simulink model "VirtualADAPT"  (Simulink 8.9)
settings: Solver=ode45  StartTime=0.0  StopTime=inf  RelTol=1e-3  EnableMultiTasking=on
PostLoadFcn:
  | FaultInjectionGUIHandle = FaultInjectionGUI;
InitFcn:
  | VirtualADAPTDataStructure
undefined symbols (not defined in the file or in its folder): ln
libraries: simulink x363
(part 21 of 26)

== system VirtualADAPT/VirtualADAPTv1/PowerStorage/Battery2Subsystem/RelayEY241/Sensor/R ==
  In : Inport
  Bond : Constant
  Computing R : Subsystem
  Fault Profile : Constant  Value=FP
  JuncDB : Constant  Value=2
  Junction Type : Constant  Value=0
  Ramp : simulink/Sources/Ramp  slope=FM  start=FT  X0=R
  Resistor Value : Constant  Value=R
  Step : Step  Time=FT  Before=R  After=(1 + FM)*R  SampleTime=0
  Out : Outport
  R : Outport  Port=2
  wires:
  Computing R/1(Out1) -> Out/1
  In/1 -> Computing R/1(In1)
  Resistor Value/1 -> Computing R/2(RNominal)
  Fault Profile/1 -> Computing R/3(Fault Profile)
  Step/1 -> Computing R/4(Step)
  Ramp/1 -> Computing R/5(Ramp)
  JuncDB/1 -> Computing R/6(JuncDB)
  Bond/1 -> Computing R/7(Bond)
  Junction Type/1 -> Computing R/8(Junction Type)
  Computing R/2(Out2) -> R/1

== system VirtualADAPT/VirtualADAPTv1/PowerStorage/Battery2Subsystem/RelayEY241/Sensor/TrueValueMod ==
  u(1) : Inport
  Function : Fcn  Expr=u(1)
  Mux : Mux  Inputs=1
  y(1) : Outport
  wires:
  u(1)/1 -> Mux/1
  Mux/1 -> Function/1
  Function/1 -> y(1)/1

== system VirtualADAPT/VirtualADAPTv1/PowerStorage/Battery2Subsystem/RelayEY241/Sensor/Value ==
  u(1) : Inport
  u(2) : Inport  Port=2
  Adder : Sum  Inputs=-  SaturateOnIntegerOverflow=on  InputSameDT=on  OutDataTypeStr=Inherit: Same as first input
  Gain : Gain  Gain=-1  SaturateOnIntegerOverflow=on  OutDataTypeStr=Inherit: Same as input  ParamDataTypeStr=Inherit: Same as input
  y(1) : Outport
  y(2) : Outport  Port=2
  O : Outport  Port=3
  wires:
  Adder/1 -> Gain/1
  u(2)/1 -> y(1)/1, O/1
  u(1)/1 -> Adder/1
  Gain/1 -> y(2)/1

== system VirtualADAPT/VirtualADAPTv1/PowerStorage/Battery2Subsystem/RelayEY244/ParallelConn/determineState ==
  In1 : Inport
  In2 : Inport  Port=2
  Fcn : Fcn  Expr=(!u(1)&&u(2)) || (u(1)&&(!u(3)))
  Memory : Memory
  Mux : Mux  Inputs=3
  Out1 : Outport
  wires:
  Memory/1 -> Mux/1
  In1/1 -> Mux/2
  In2/1 -> Mux/3
  Mux/1 -> Fcn/1
  Fcn/1 -> Memory/1, Out1/1

== system VirtualADAPT/VirtualADAPTv1/PowerStorage/Battery2Subsystem/RelayEY244/Relay/determineState ==
  In1 : Inport
  In2 : Inport  Port=2
  Fcn : Fcn  Expr=(!u(1)&&u(2)) || (u(1)&&(!u(3)))
  Memory : Memory
  Mux : Mux  Inputs=3
  Out1 : Outport
  wires:
  Memory/1 -> Mux/1
  In1/1 -> Mux/2
  In2/1 -> Mux/3
  Mux/1 -> Fcn/1
  Fcn/1 -> Memory/1, Out1/1

== system VirtualADAPT/VirtualADAPTv1/PowerStorage/Battery2Subsystem/RelayEY244/Sensor/MSe ==
  F : Inport
  Se : Inport  Port=2
  Bias : Step  Time=FT  After=FM  SampleTime=0
  Fault Inject : Subsystem
  Fault Profile : Constant  Value=FP
  Mult2 : Product  SaturateOnIntegerOverflow=on  RndMeth=Zero  InputSameDT=on  OutDataTypeStr=Inherit: Same as first input
  Multiply : Product  SaturateOnIntegerOverflow=on  RndMeth=Zero  InputSameDT=on  OutDataTypeStr=Inherit: Same as first input
  Ramp : simulink/Sources/Ramp  slope=FM  start=FT  X0=0
  Step : Step  Time=FT  Before=1  After=(1 + FM)  SampleTime=0
  StuckAt : Step  Time=FT  Before=1  After=0  SampleTime=0
  StuckAt2 : Step  Time=FT  After=FM  SampleTime=0
  Sum : Sum  SaturateOnIntegerOverflow=on  InputSameDT=on  OutDataTypeStr=Inherit: Same as first input
  Sum2 : Sum  SaturateOnIntegerOverflow=on  InputSameDT=on  OutDataTypeStr=Inherit: Same as first input
  Sum3 : Sum  SaturateOnIntegerOverflow=on  InputSameDT=on  OutDataTypeStr=Inherit: Same as first input
  E : Outport
  wires:
  Fault Inject/1(Out1) -> E/1
  Se/1 -> Fault Inject/1(Nominal), Multiply/1, Sum/1, Sum2/1, Mult2/1
  Fault Profile/1 -> Fault Inject/2(Fault Profile)
  Step/1 -> Multiply/2
  Ramp/1 -> Sum/2
  Multiply/1 -> Fault Inject/3(Step)
  Sum/1 -> Fault Inject/4(Ramp)
  Bias/1 -> Sum2/2
  Sum2/1 -> Fault Inject/5(Bias)
  StuckAt/1 -> Mult2/2
  Mult2/1 -> Sum3/1
  StuckAt2/1 -> Sum3/2
  Sum3/1 -> Fault Inject/6(stuckAt)

== system VirtualADAPT/VirtualADAPTv1/PowerStorage/Battery2Subsystem/RelayEY244/Sensor/R ==
  In : Inport
  Bond : Constant
  Computing R : Subsystem
  Fault Profile : Constant  Value=FP
  JuncDB : Constant  Value=2
  Junction Type : Constant  Value=0
  Ramp : simulink/Sources/Ramp  slope=FM  start=FT  X0=R
  Resistor Value : Constant  Value=R
  Step : Step  Time=FT  Before=R  After=(1 + FM)*R  SampleTime=0
  Out : Outport
  R : Outport  Port=2
  wires:
  Computing R/1(Out1) -> Out/1
  In/1 -> Computing R/1(In1)
  Resistor Value/1 -> Computing R/2(RNominal)
  Fault Profile/1 -> Computing R/3(Fault Profile)
  Step/1 -> Computing R/4(Step)
  Ramp/1 -> Computing R/5(Ramp)
  JuncDB/1 -> Computing R/6(JuncDB)
  Bond/1 -> Computing R/7(Bond)
  Junction Type/1 -> Computing R/8(Junction Type)
  Computing R/2(Out2) -> R/1

== system VirtualADAPT/VirtualADAPTv1/PowerStorage/Battery2Subsystem/RelayEY244/Sensor/TrueValueMod ==
  u(1) : Inport
  Function : Fcn  Expr=u(1)
  Mux : Mux  Inputs=1
  y(1) : Outport
  wires:
  u(1)/1 -> Mux/1
  Mux/1 -> Function/1
  Function/1 -> y(1)/1

== system VirtualADAPT/VirtualADAPTv1/PowerStorage/Battery2Subsystem/RelayEY244/Sensor/Value ==
  u(1) : Inport
  u(2) : Inport  Port=2
  Adder : Sum  Inputs=-  SaturateOnIntegerOverflow=on  InputSameDT=on  OutDataTypeStr=Inherit: Same as first input
  Gain : Gain  Gain=-1  SaturateOnIntegerOverflow=on  OutDataTypeStr=Inherit: Same as input  ParamDataTypeStr=Inherit: Same as input
  y(1) : Outport
  y(2) : Outport  Port=2
  O : Outport  Port=3
  wires:
  Adder/1 -> Gain/1
  u(2)/1 -> y(1)/1, O/1
  u(1)/1 -> Adder/1
  Gain/1 -> y(2)/1

== system VirtualADAPT/VirtualADAPTv1/PowerStorage/Battery2Subsystem/SensorE235/MSe/Fault Inject ==
  Nominal : Inport
  Fault Profile : Inport  Port=2
  Step : Inport  Port=3
  Ramp : Inport  Port=4
  Bias : Inport  Port=5
  stuckAt : Inport  Port=6
  Switch1 : MultiPortSwitch  Inputs=5  SaturateOnIntegerOverflow=on  InputSameDT=on
  Out1 : Outport
  wires:
  Nominal/1 -> Switch1/2
  Fault Profile/1 -> Switch1/1
  Step/1 -> Switch1/3
  Ramp/1 -> Switch1/4
  Bias/1 -> Switch1/5
  stuckAt/1 -> Switch1/6
  Switch1/1 -> Out1/1

== system VirtualADAPT/VirtualADAPTv1/PowerStorage/Battery2Subsystem/SensorE235/R/Computing R ==
  In1 : Inport
  RNominal : Inport  Port=2
  Fault Profile : Inport  Port=3
  Step : Inport  Port=4
  Ramp : Inport  Port=5
  JuncDB : Inport  Port=6
  Bond : Inport  Port=7
  Junction Type : Inport  Port=8
  CalcCausality : Fcn  Expr=(u(1) == u(2) && u(3) ==1) || (u(1) !=u(2) && u(3) == 0)
  CalcOutput : Fcn  Expr=(u(1)*u(2)^u(3))
  MinusOne : Constant  Value=-1
  Mux1 : Mux  Inputs=3
  Mux2 : Mux  Inputs=3
  One : Constant
  Switch1 : MultiPortSwitch  SaturateOnIntegerOverflow=on  InputSameDT=on
  Switch2 : MultiPortSwitch  DataPortOrder=Zero-based contiguous  Inputs=2  zeroidx=on  SaturateOnIntegerOverflow=on  InputSameDT=on
  Out1 : Outport
  Out2 : Outport  Port=2
  wires:
  In1/1 -> Mux2/1
  Fault Profile/1 -> Switch1/1
  RNominal/1 -> Switch1/2
  Step/1 -> Switch1/3
  Ramp/1 -> Switch1/4
  Switch1/1 -> Out2/1, Mux2/2
  JuncDB/1 -> Mux1/1
  Bond/1 -> Mux1/2
  Junction Type/1 -> Mux1/3
  Mux1/1 -> CalcCausality/1
  CalcCausality/1 -> Switch2/1
  One/1 -> Switch2/2
  MinusOne/1 -> Switch2/3
  Switch2/1 -> Mux2/3
  Mux2/1 -> CalcOutput/1
  CalcOutput/1 -> Out1/1

== system VirtualADAPT/VirtualADAPTv1/PowerStorage/Battery2Subsystem/SensorE240/MSe/Fault Inject ==
  Nominal : Inport
  Fault Profile : Inport  Port=2
  Step : Inport  Port=3
  Ramp : Inport  Port=4
  Bias : Inport  Port=5
  stuckAt : Inport  Port=6
  Switch1 : MultiPortSwitch  Inputs=5  SaturateOnIntegerOverflow=on  InputSameDT=on
  Out1 : Outport
  wires:
  Nominal/1 -> Switch1/2
  Fault Profile/1 -> Switch1/1
  Step/1 -> Switch1/3
  Ramp/1 -> Switch1/4
  Bias/1 -> Switch1/5
  stuckAt/1 -> Switch1/6
  Switch1/1 -> Out1/1

== system VirtualADAPT/VirtualADAPTv1/PowerStorage/Battery2Subsystem/SensorE240/R/Computing R ==
  In1 : Inport
  RNominal : Inport  Port=2
  Fault Profile : Inport  Port=3
  Step : Inport  Port=4
  Ramp : Inport  Port=5
  JuncDB : Inport  Port=6
  Bond : Inport  Port=7
  Junction Type : Inport  Port=8
  CalcCausality : Fcn  Expr=(u(1) == u(2) && u(3) ==1) || (u(1) !=u(2) && u(3) == 0)
  CalcOutput : Fcn  Expr=(u(1)*u(2)^u(3))
  MinusOne : Constant  Value=-1
  Mux1 : Mux  Inputs=3
  Mux2 : Mux  Inputs=3
  One : Constant
  Switch1 : MultiPortSwitch  SaturateOnIntegerOverflow=on  InputSameDT=on
  Switch2 : MultiPortSwitch  DataPortOrder=Zero-based contiguous  Inputs=2  zeroidx=on  SaturateOnIntegerOverflow=on  InputSameDT=on
  Out1 : Outport
  Out2 : Outport  Port=2
  wires:
  In1/1 -> Mux2/1
  Fault Profile/1 -> Switch1/1
  RNominal/1 -> Switch1/2
  Step/1 -> Switch1/3
  Ramp/1 -> Switch1/4
  Switch1/1 -> Out2/1, Mux2/2
  JuncDB/1 -> Mux1/1
  Bond/1 -> Mux1/2
  Junction Type/1 -> Mux1/3
  Mux1/1 -> CalcCausality/1
  CalcCausality/1 -> Switch2/1
  One/1 -> Switch2/2
  MinusOne/1 -> Switch2/3
  Switch2/1 -> Mux2/3
  Mux2/1 -> CalcOutput/1
  CalcOutput/1 -> Out1/1

== system VirtualADAPT/VirtualADAPTv1/PowerStorage/Battery2Subsystem/SensorE242/MSe/Fault Inject ==
  Nominal : Inport
  Fault Profile : Inport  Port=2
  Step : Inport  Port=3
  Ramp : Inport  Port=4
  Bias : Inport  Port=5
  stuckAt : Inport  Port=6
  Switch1 : MultiPortSwitch  Inputs=5  SaturateOnIntegerOverflow=on  InputSameDT=on
  Out1 : Outport
  wires:
  Nominal/1 -> Switch1/2
  Fault Profile/1 -> Switch1/1
  Step/1 -> Switch1/3
  Ramp/1 -> Switch1/4
  Bias/1 -> Switch1/5
  stuckAt/1 -> Switch1/6
  Switch1/1 -> Out1/1

== system VirtualADAPT/VirtualADAPTv1/PowerStorage/Battery2Subsystem/SensorE242/R/Computing R ==
  In1 : Inport
  RNominal : Inport  Port=2
  Fault Profile : Inport  Port=3
  Step : Inport  Port=4
  Ramp : Inport  Port=5
  JuncDB : Inport  Port=6
  Bond : Inport  Port=7
  Junction Type : Inport  Port=8
  CalcCausality : Fcn  Expr=(u(1) == u(2) && u(3) ==1) || (u(1) !=u(2) && u(3) == 0)
  CalcOutput : Fcn  Expr=(u(1)*u(2)^u(3))
  MinusOne : Constant  Value=-1
  Mux1 : Mux  Inputs=3
  Mux2 : Mux  Inputs=3
  One : Constant
  Switch1 : MultiPortSwitch  SaturateOnIntegerOverflow=on  InputSameDT=on
  Switch2 : MultiPortSwitch  DataPortOrder=Zero-based contiguous  Inputs=2  zeroidx=on  SaturateOnIntegerOverflow=on  InputSameDT=on
  Out1 : Outport
  Out2 : Outport  Port=2
  wires:
  In1/1 -> Mux2/1
  Fault Profile/1 -> Switch1/1
  RNominal/1 -> Switch1/2
  Step/1 -> Switch1/3
  Ramp/1 -> Switch1/4
  Switch1/1 -> Out2/1, Mux2/2
  JuncDB/1 -> Mux1/1
  Bond/1 -> Mux1/2
  Junction Type/1 -> Mux1/3
  Mux1/1 -> CalcCausality/1
  CalcCausality/1 -> Switch2/1
  One/1 -> Switch2/2
  MinusOne/1 -> Switch2/3
  Switch2/1 -> Mux2/3
  Mux2/1 -> CalcOutput/1
  CalcOutput/1 -> Out1/1

== system VirtualADAPT/VirtualADAPTv1/PowerStorage/Battery2Subsystem/SensorISH236/MSe/Fault Inject ==
  Nominal : Inport
  Fault Profile : Inport  Port=2
  Step : Inport  Port=3
  Ramp : Inport  Port=4
  Bias : Inport  Port=5
  stuckAt : Inport  Port=6
  Switch1 : MultiPortSwitch  Inputs=5  SaturateOnIntegerOverflow=on  InputSameDT=on
  Out1 : Outport
  wires:
  Nominal/1 -> Switch1/2
  Fault Profile/1 -> Switch1/1
  Step/1 -> Switch1/3
  Ramp/1 -> Switch1/4
  Bias/1 -> Switch1/5
  stuckAt/1 -> Switch1/6
  Switch1/1 -> Out1/1

== system VirtualADAPT/VirtualADAPTv1/PowerStorage/Battery2Subsystem/SensorISH236/R/Computing R ==
  In1 : Inport
  RNominal : Inport  Port=2
  Fault Profile : Inport  Port=3
  Step : Inport  Port=4
  Ramp : Inport  Port=5
  JuncDB : Inport  Port=6
  Bond : Inport  Port=7
  Junction Type : Inport  Port=8
  CalcCausality : Fcn  Expr=(u(1) == u(2) && u(3) ==1) || (u(1) !=u(2) && u(3) == 0)
  CalcOutput : Fcn  Expr=(u(1)*u(2)^u(3))
  MinusOne : Constant  Value=-1
  Mux1 : Mux  Inputs=3
  Mux2 : Mux  Inputs=3
  One : Constant
  Switch1 : MultiPortSwitch  SaturateOnIntegerOverflow=on  InputSameDT=on
  Switch2 : MultiPortSwitch  DataPortOrder=Zero-based contiguous  Inputs=2  zeroidx=on  SaturateOnIntegerOverflow=on  InputSameDT=on
  Out1 : Outport
  Out2 : Outport  Port=2
  wires:
  In1/1 -> Mux2/1
  Fault Profile/1 -> Switch1/1
  RNominal/1 -> Switch1/2
  Step/1 -> Switch1/3
  Ramp/1 -> Switch1/4
  Switch1/1 -> Out2/1, Mux2/2
  JuncDB/1 -> Mux1/1
  Bond/1 -> Mux1/2
  Junction Type/1 -> Mux1/3
  Mux1/1 -> CalcCausality/1
  CalcCausality/1 -> Switch2/1
  One/1 -> Switch2/2
  MinusOne/1 -> Switch2/3
  Switch2/1 -> Mux2/3
  Mux2/1 -> CalcOutput/1
  CalcOutput/1 -> Out1/1

== system VirtualADAPT/VirtualADAPTv1/PowerStorage/Battery2Subsystem/SensorIT240/MSe/Fault Inject ==
  Nominal : Inport
  Fault Profile : Inport  Port=2
  Step : Inport  Port=3
  Ramp : Inport  Port=4
  Bias : Inport  Port=5
  stuckAt : Inport  Port=6
  Switch1 : MultiPortSwitch  Inputs=5  SaturateOnIntegerOverflow=on  InputSameDT=on
  Out1 : Outport
  wires:
  Nominal/1 -> Switch1/2
  Fault Profile/1 -> Switch1/1
  Step/1 -> Switch1/3
  Ramp/1 -> Switch1/4
  Bias/1 -> Switch1/5
  stuckAt/1 -> Switch1/6
  Switch1/1 -> Out1/1

== system VirtualADAPT/VirtualADAPTv1/PowerStorage/Battery2Subsystem/SensorIT240/R/Computing R ==
  In1 : Inport
  RNominal : Inport  Port=2
  Fault Profile : Inport  Port=3
  Step : Inport  Port=4
  Ramp : Inport  Port=5
  JuncDB : Inport  Port=6
  Bond : Inport  Port=7
  Junction Type : Inport  Port=8
  CalcCausality : Fcn  Expr=(u(1) == u(2) && u(3) ==1) || (u(1) !=u(2) && u(3) == 0)
  CalcOutput : Fcn  Expr=(u(1)*u(2)^u(3))
  MinusOne : Constant  Value=-1
  Mux1 : Mux  Inputs=3
  Mux2 : Mux  Inputs=3
  One : Constant
  Switch1 : MultiPortSwitch  SaturateOnIntegerOverflow=on  InputSameDT=on
  Switch2 : MultiPortSwitch  DataPortOrder=Zero-based contiguous  Inputs=2  zeroidx=on  SaturateOnIntegerOverflow=on  InputSameDT=on
  Out1 : Outport
  Out2 : Outport  Port=2
  wires:
  In1/1 -> Mux2/1
  Fault Profile/1 -> Switch1/1
  RNominal/1 -> Switch1/2
  Step/1 -> Switch1/3
  Ramp/1 -> Switch1/4
  Switch1/1 -> Out2/1, Mux2/2
  JuncDB/1 -> Mux1/1
  Bond/1 -> Mux1/2
  Junction Type/1 -> Mux1/3
  Mux1/1 -> CalcCausality/1
  CalcCausality/1 -> Switch2/1
  One/1 -> Switch2/2
  MinusOne/1 -> Switch2/3
  Switch2/1 -> Mux2/3
  Mux2/1 -> CalcOutput/1
  CalcOutput/1 -> Out1/1

== system VirtualADAPT/VirtualADAPTv1/PowerStorage/Battery2Subsystem/SensorTE228/MSe/Fault Inject ==
  Nominal : Inport
  Fault Profile : Inport  Port=2
  Step : Inport  Port=3
  Ramp : Inport  Port=4
  Bias : Inport  Port=5
  stuckAt : Inport  Port=6
  Switch1 : MultiPortSwitch  Inputs=5  SaturateOnIntegerOverflow=on  InputSameDT=on
  Out1 : Outport
  wires:
  Nominal/1 -> Switch1/2
  Fault Profile/1 -> Switch1/1
  Step/1 -> Switch1/3
  Ramp/1 -> Switch1/4
  Bias/1 -> Switch1/5
  stuckAt/1 -> Switch1/6
  Switch1/1 -> Out1/1

== system VirtualADAPT/VirtualADAPTv1/PowerStorage/Battery2Subsystem/SensorTE228/R/Computing R ==
  In1 : Inport
  RNominal : Inport  Port=2
  Fault Profile : Inport  Port=3
  Step : Inport  Port=4
  Ramp : Inport  Port=5
  JuncDB : Inport  Port=6
  Bond : Inport  Port=7
  Junction Type : Inport  Port=8
  CalcCausality : Fcn  Expr=(u(1) == u(2) && u(3) ==1) || (u(1) !=u(2) && u(3) == 0)
  CalcOutput : Fcn  Expr=(u(1)*u(2)^u(3))
  MinusOne : Constant  Value=-1
  Mux1 : Mux  Inputs=3
  Mux2 : Mux  Inputs=3
  One : Constant
  Switch1 : MultiPortSwitch  SaturateOnIntegerOverflow=on  InputSameDT=on
  Switch2 : MultiPortSwitch  DataPortOrder=Zero-based contiguous  Inputs=2  zeroidx=on  SaturateOnIntegerOverflow=on  InputSameDT=on
  Out1 : Outport
  Out2 : Outport  Port=2
  wires:
  In1/1 -> Mux2/1
  Fault Profile/1 -> Switch1/1
  RNominal/1 -> Switch1/2
  Step/1 -> Switch1/3
  Ramp/1 -> Switch1/4
  Switch1/1 -> Out2/1, Mux2/2
  JuncDB/1 -> Mux1/1
  Bond/1 -> Mux1/2
  Junction Type/1 -> Mux1/3
  Mux1/1 -> CalcCausality/1
  CalcCausality/1 -> Switch2/1
  One/1 -> Switch2/2
  MinusOne/1 -> Switch2/3
  Switch2/1 -> Mux2/3
  Mux2/1 -> CalcOutput/1
  CalcOutput/1 -> Out1/1

== system VirtualADAPT/VirtualADAPTv1/PowerStorage/Battery2Subsystem/SensorTE228/TempBias/Fault Inject ==
  Nominal : Inport
  Fault Profile : Inport  Port=2
  Step : Inport  Port=3
  Ramp : Inport  Port=4
  Bias : Inport  Port=5
  stuckAt : Inport  Port=6
  Switch1 : MultiPortSwitch  Inputs=5  SaturateOnIntegerOverflow=on  InputSameDT=on
  Out1 : Outport
  wires:
  Nominal/1 -> Switch1/2
  Fault Profile/1 -> Switch1/1
  Step/1 -> Switch1/3
  Ramp/1 -> Switch1/4
  Bias/1 -> Switch1/5
  stuckAt/1 -> Switch1/6
  Switch1/1 -> Out1/1

== system VirtualADAPT/VirtualADAPTv1/PowerStorage/Battery2Subsystem/SensorTE229/MSe/Fault Inject ==
  Nominal : Inport
  Fault Profile : Inport  Port=2
  Step : Inport  Port=3
  Ramp : Inport  Port=4
  Bias : Inport  Port=5
  stuckAt : Inport  Port=6
  Switch1 : MultiPortSwitch  Inputs=5  SaturateOnIntegerOverflow=on  InputSameDT=on
  Out1 : Outport
  wires:
  Nominal/1 -> Switch1/2
  Fault Profile/1 -> Switch1/1
  Step/1 -> Switch1/3
  Ramp/1 -> Switch1/4
  Bias/1 -> Switch1/5
  stuckAt/1 -> Switch1/6
  Switch1/1 -> Out1/1

== system VirtualADAPT/VirtualADAPTv1/PowerStorage/Battery2Subsystem/SensorTE229/R/Computing R ==
  In1 : Inport
  RNominal : Inport  Port=2
  Fault Profile : Inport  Port=3
  Step : Inport  Port=4
  Ramp : Inport  Port=5
  JuncDB : Inport  Port=6
  Bond : Inport  Port=7
  Junction Type : Inport  Port=8
  CalcCausality : Fcn  Expr=(u(1) == u(2) && u(3) ==1) || (u(1) !=u(2) && u(3) == 0)
  CalcOutput : Fcn  Expr=(u(1)*u(2)^u(3))
  MinusOne : Constant  Value=-1
  Mux1 : Mux  Inputs=3
  Mux2 : Mux  Inputs=3
  One : Constant
  Switch1 : MultiPortSwitch  SaturateOnIntegerOverflow=on  InputSameDT=on
  Switch2 : MultiPortSwitch  DataPortOrder=Zero-based contiguous  Inputs=2  zeroidx=on  SaturateOnIntegerOverflow=on  InputSameDT=on
  Out1 : Outport
  Out2 : Outport  Port=2
  wires:
  In1/1 -> Mux2/1
  Fault Profile/1 -> Switch1/1
  RNominal/1 -> Switch1/2
  Step/1 -> Switch1/3
  Ramp/1 -> Switch1/4
  Switch1/1 -> Out2/1, Mux2/2
  JuncDB/1 -> Mux1/1
  Bond/1 -> Mux1/2
  Junction Type/1 -> Mux1/3
  Mux1/1 -> CalcCausality/1
  CalcCausality/1 -> Switch2/1
  One/1 -> Switch2/2
  MinusOne/1 -> Switch2/3
  Switch2/1 -> Mux2/3
  Mux2/1 -> CalcOutput/1
  CalcOutput/1 -> Out1/1

== system VirtualADAPT/VirtualADAPTv1/PowerStorage/Battery2Subsystem/SensorTE229/TempBias/Fault Inject ==
  Nominal : Inport
  Fault Profile : Inport  Port=2
  Step : Inport  Port=3
  Ramp : Inport  Port=4
  Bias : Inport  Port=5
  stuckAt : Inport  Port=6
  Switch1 : MultiPortSwitch  Inputs=5  SaturateOnIntegerOverflow=on  InputSameDT=on
  Out1 : Outport
  wires:
  Nominal/1 -> Switch1/2
  Fault Profile/1 -> Switch1/1
  Step/1 -> Switch1/3
  Ramp/1 -> Switch1/4
  Bias/1 -> Switch1/5
  stuckAt/1 -> Switch1/6
  Switch1/1 -> Out1/1

== system VirtualADAPT/VirtualADAPTv1/PowerStorage/RelayEY160/Sensor/MSe/Fault Inject ==
  Nominal : Inport
  Fault Profile : Inport  Port=2
  Step : Inport  Port=3
  Ramp : Inport  Port=4
  Bias : Inport  Port=5
  stuckAt : Inport  Port=6
  Switch1 : MultiPortSwitch  Inputs=5  SaturateOnIntegerOverflow=on  InputSameDT=on
  Out1 : Outport
  wires:
  Nominal/1 -> Switch1/2
  Fault Profile/1 -> Switch1/1
  Step/1 -> Switch1/3
  Ramp/1 -> Switch1/4
  Bias/1 -> Switch1/5
  stuckAt/1 -> Switch1/6
  Switch1/1 -> Out1/1

== system VirtualADAPT/VirtualADAPTv1/PowerStorage/RelayEY160/Sensor/R/Computing R ==
  In1 : Inport
  RNominal : Inport  Port=2
  Fault Profile : Inport  Port=3
  Step : Inport  Port=4
  Ramp : Inport  Port=5
  JuncDB : Inport  Port=6
  Bond : Inport  Port=7
  Junction Type : Inport  Port=8
  CalcCausality : Fcn  Expr=(u(1) == u(2) && u(3) ==1) || (u(1) !=u(2) && u(3) == 0)
  CalcOutput : Fcn  Expr=(u(1)*u(2)^u(3))
  MinusOne : Constant  Value=-1
  Mux1 : Mux  Inputs=3
  Mux2 : Mux  Inputs=3
  One : Constant
  Switch1 : MultiPortSwitch  SaturateOnIntegerOverflow=on  InputSameDT=on
  Switch2 : MultiPortSwitch  DataPortOrder=Zero-based contiguous  Inputs=2  zeroidx=on  SaturateOnIntegerOverflow=on  InputSameDT=on
  Out1 : Outport
  Out2 : Outport  Port=2
  wires:
  In1/1 -> Mux2/1
  Fault Profile/1 -> Switch1/1
  RNominal/1 -> Switch1/2
  Step/1 -> Switch1/3
  Ramp/1 -> Switch1/4
  Switch1/1 -> Out2/1, Mux2/2
  JuncDB/1 -> Mux1/1
  Bond/1 -> Mux1/2
  Junction Type/1 -> Mux1/3
  Mux1/1 -> CalcCausality/1
  CalcCausality/1 -> Switch2/1
  One/1 -> Switch2/2
  MinusOne/1 -> Switch2/3
  Switch2/1 -> Mux2/3
  Mux2/1 -> CalcOutput/1
  CalcOutput/1 -> Out1/1

== system VirtualADAPT/VirtualADAPTv1/PowerStorage/RelayEY260/Sensor/MSe/Fault Inject ==
  Nominal : Inport
  Fault Profile : Inport  Port=2
  Step : Inport  Port=3
  Ramp : Inport  Port=4
  Bias : Inport  Port=5
  stuckAt : Inport  Port=6
  Switch1 : MultiPortSwitch  Inputs=5  SaturateOnIntegerOverflow=on  InputSameDT=on
  Out1 : Outport
  wires:
  Nominal/1 -> Switch1/2
  Fault Profile/1 -> Switch1/1
  Step/1 -> Switch1/3
  Ramp/1 -> Switch1/4
  Bias/1 -> Switch1/5
  stuckAt/1 -> Switch1/6
  Switch1/1 -> Out1/1

== system VirtualADAPT/VirtualADAPTv1/PowerStorage/RelayEY260/Sensor/R/Computing R ==
  In1 : Inport
  RNominal : Inport  Port=2
  Fault Profile : Inport  Port=3
  Step : Inport  Port=4
  Ramp : Inport  Port=5
  JuncDB : Inport  Port=6
  Bond : Inport  Port=7
  Junction Type : Inport  Port=8
  CalcCausality : Fcn  Expr=(u(1) == u(2) && u(3) ==1) || (u(1) !=u(2) && u(3) == 0)
  CalcOutput : Fcn  Expr=(u(1)*u(2)^u(3))
  MinusOne : Constant  Value=-1
  Mux1 : Mux  Inputs=3
  Mux2 : Mux  Inputs=3
  One : Constant
  Switch1 : MultiPortSwitch  SaturateOnIntegerOverflow=on  InputSameDT=on
  Switch2 : MultiPortSwitch  DataPortOrder=Zero-based contiguous  Inputs=2  zeroidx=on  SaturateOnIntegerOverflow=on  InputSameDT=on
  Out1 : Outport
  Out2 : Outport  Port=2
  wires:
  In1/1 -> Mux2/1
  Fault Profile/1 -> Switch1/1
  RNominal/1 -> Switch1/2
  Step/1 -> Switch1/3
  Ramp/1 -> Switch1/4
  Switch1/1 -> Out2/1, Mux2/2
  JuncDB/1 -> Mux1/1
  Bond/1 -> Mux1/2
  Junction Type/1 -> Mux1/3
  Mux1/1 -> CalcCausality/1
  CalcCausality/1 -> Switch2/1
  One/1 -> Switch2/2
  MinusOne/1 -> Switch2/3
  Switch2/1 -> Mux2/3
  Mux2/1 -> CalcOutput/1
  CalcOutput/1 -> Out1/1

== system VirtualADAPT/VirtualADAPTv1/PowerDistribution/ACLoadBank1/L1A/LGT6/LightBulb1/ComputePower ==
  u(1) : Inport
  u(2) : Inport  Port=2
  Function : Fcn  Expr=abs(u(1)*u(2))
  Mux : Mux  Inputs=2
  y(1) : Outport
  wires:
  u(1)/1 -> Mux/1
  u(2)/1 -> Mux/2
  Mux/1 -> Function/1
  Function/1 -> y(1)/1

== system VirtualADAPT/VirtualADAPTv1/PowerDistribution/ACLoadBank1/L1A/LGT6/LightBulb1/I ==
  u(1) : Inport
  u(2) : Inport  Port=2
  Adder : Sum  Inputs=+  SaturateOnIntegerOverflow=on  InputSameDT=on  OutDataTypeStr=Inherit: Same as first input
  Gain : Gain  SaturateOnIntegerOverflow=on  OutDataTypeStr=Inherit: Same as input  ParamDataTypeStr=Inherit: Same as input
  y(1) : Outport
  y(2) : Outport  Port=2
  O : Outport  Port=3
  wires:
  Adder/1 -> Gain/1
  Gain/1 -> y(1)/1
  u(1)/1 -> y(2)/1, O/1
  u(2)/1 -> Adder/1

== system VirtualADAPT/VirtualADAPTv1/PowerDistribution/ACLoadBank1/L1A/LGT6/LightBulb1/R ==
  In : Inport
  Bond : Constant  Value=2
  Computing R : Subsystem
  Fault Profile : Constant  Value=FP
  JuncDB : Constant
  Junction Type : Constant  Value=0
  Ramp : simulink/Sources/Ramp  slope=FM  start=FT  X0=R
  Resistor Value : Constant  Value=R
  Step : Step  Time=FT  Before=R  After=120.5/(FM+120.5/R)  SampleTime=0
  Out : Outport
  R : Outport  Port=2
  wires:
  Computing R/1(Out1) -> Out/1
  In/1 -> Computing R/1(In1)
  Resistor Value/1 -> Computing R/2(RNominal)
  Fault Profile/1 -> Computing R/3(Fault Profile)
  Step/1 -> Computing R/4(Step)
  Ramp/1 -> Computing R/5(Ramp)
  JuncDB/1 -> Computing R/6(JuncDB)
  Bond/1 -> Computing R/7(Bond)
  Junction Type/1 -> Computing R/8(Junction Type)
  Computing R/2(Out2) -> R/1

== system VirtualADAPT/VirtualADAPTv1/PowerDistribution/ACLoadBank1/L1A/LGT6/LightBulb1/TemperatureModel ==
  AmbientTemp : Inport
  Power : Inport  Port=2
  AmbTemp : Subsystem  mask{FP=Nominal, FT=0, FM=0}
      mask prompts: FP "Fault Profile:"; FT "Fault Injection Time:"; FM "Fault Magnitude/Slope/Bias/StuckAtValue"
      mask TypeOptions FP: Nominal | Abrupt | Incipient | Bias | StuckAt
      mask Display: image(imread('MSe.png','BackgroundColor',[1 1 1]))
  Ctheta : Subsystem  mask{C=51.711, IC=22, FP=Nominal, FT=0, FM=0}
      mask prompts: C "Value of C:"; IC "Initial Condition:"; FP "Fault Profile:"; FT "Fault Injection Time:"; FM "Fault Magnitude/Slope"
      mask TypeOptions FP: Nominal | Abrupt | Incipient
      mask Display: image(imread('C.png','BackgroundColor',[1 1 1]))
  ModAmbTemp : Subsystem
      mask Display: image(imread('fx.png','BackgroundColor',[1 1 1]))
  ModPs : Subsystem
      mask Display: image(imread('fx.png','BackgroundColor',[1 1 1]))
  OJ : Subsystem
      mask Display: image(imread('1.png','BackgroundColor',[1 1 1]))
  Rtheta : Subsystem  mask{R=2.3686, FP=Nominal, FT=0, FM=0}
      mask prompts: R "Value of R:"; FP "Fault Profile:"; FT "Fault Injection Time:"; FM "Fault Magnitude/Slope"
      mask TypeOptions FP: Nominal | Abrupt | Incipient
      mask Display: image(imread('R.png','BackgroundColor',[1 1 1]))
  SfPs : Subsystem  mask{FP=Nominal, FT=0, FM=0}
      mask prompts: FP "Fault Profile:"; FT "Fault Injection Time:"; FM "Fault Magnitude/Slope/Bias/StuckAtValue"
      mask TypeOptions FP: Nominal | Abrupt | Incipient | Bias | StuckAt
      mask Display: image(imread('MSf.png','BackgroundColor',[1 1 1]))
  theta : Subsystem
      mask Display: image(imread('0.png','BackgroundColor',[1 1 1]))
  Temp : Outport
  wires:
  theta/1(y(1)) -> Ctheta/1(F)
  Ctheta/1(E) -> theta/1(u(1))
  OJ/1(y(1)) -> Rtheta/1(In)
  Rtheta/1(Out) -> OJ/1(u(1))
  theta/2(y(2)) -> OJ/2(u(2))
  OJ/2(y(2)) -> theta/2(u(2))
  SfPs/1(F) -> theta/3(u(3))
  theta/3(y(3)) -> SfPs/1(E)
  AmbTemp/1(E) -> OJ/3(u(3))
  OJ/3(y(3)) -> AmbTemp/1(F)
  ModAmbTemp/1(y(1)) -> AmbTemp/2(Se)
  ModPs/1(y(1)) -> SfPs/2(Sf)
  Power/1 -> ModPs/1(u(1))
  AmbientTemp/1 -> ModAmbTemp/1(u(1))
  theta/4(O) -> Temp/1

== system VirtualADAPT/VirtualADAPTv1/PowerDistribution/ACLoadBank1/L1A/LGT6/LightBulb1/V ==
  u(1) : Inport
  u(2) : Inport  Port=2
  Adder : Sum  Inputs=-  SaturateOnIntegerOverflow=on  InputSameDT=on  OutDataTypeStr=Inherit: Same as first input
  Gain : Gain  Gain=-1  SaturateOnIntegerOverflow=on  OutDataTypeStr=Inherit: Same as input  ParamDataTypeStr=Inherit: Same as input
  y(1) : Outport
  y(2) : Outport  Port=2
  O : Outport  Port=3
  wires:
  Adder/1 -> Gain/1
  Gain/1 -> y(1)/1
  u(1)/1 -> y(2)/1, O/1
  u(2)/1 -> Adder/1

== system VirtualADAPT/VirtualADAPTv1/PowerDistribution/ACLoadBank1/L1A/LGT6/LightBulb2/ComputePower ==
  u(1) : Inport
  u(2) : Inport  Port=2
  Function : Fcn  Expr=abs(u(2)*u(1))
  Mux : Mux  Inputs=2
  y(1) : Outport
  wires:
  u(1)/1 -> Mux/1
  u(2)/1 -> Mux/2
  Mux/1 -> Function/1
  Function/1 -> y(1)/1

== system VirtualADAPT/VirtualADAPTv1/PowerDistribution/ACLoadBank1/L1A/LGT6/LightBulb2/I ==
  u(1) : Inport
  u(2) : Inport  Port=2
  Adder : Sum  Inputs=+  SaturateOnIntegerOverflow=on  InputSameDT=on  OutDataTypeStr=Inherit: Same as first input
  Gain : Gain  SaturateOnIntegerOverflow=on  OutDataTypeStr=Inherit: Same as input  ParamDataTypeStr=Inherit: Same as input
  y(1) : Outport
  y(2) : Outport  Port=2
  O : Outport  Port=3
  wires:
  Adder/1 -> Gain/1
  Gain/1 -> y(1)/1
  u(1)/1 -> y(2)/1, O/1
  u(2)/1 -> Adder/1

== system VirtualADAPT/VirtualADAPTv1/PowerDistribution/ACLoadBank1/L1A/LGT6/LightBulb2/R ==
  In : Inport
  Bond : Constant  Value=2
  Computing R : Subsystem
  Fault Profile : Constant  Value=FP
  JuncDB : Constant
  Junction Type : Constant  Value=0
  Ramp : simulink/Sources/Ramp  slope=FM  start=FT  X0=R
  Resistor Value : Constant  Value=R
  Step : Step  Time=FT  Before=R  After=120.5/(FM+120.5/R)  SampleTime=0
  Out : Outport
  R : Outport  Port=2
  wires:
  Computing R/1(Out1) -> Out/1
  In/1 -> Computing R/1(In1)
  Resistor Value/1 -> Computing R/2(RNominal)
  Fault Profile/1 -> Computing R/3(Fault Profile)
  Step/1 -> Computing R/4(Step)
  Ramp/1 -> Computing R/5(Ramp)
  JuncDB/1 -> Computing R/6(JuncDB)
  Bond/1 -> Computing R/7(Bond)
  Junction Type/1 -> Computing R/8(Junction Type)
  Computing R/2(Out2) -> R/1

== system VirtualADAPT/VirtualADAPTv1/PowerDistribution/ACLoadBank1/L1A/LGT6/LightBulb2/TemperatureModel ==
  AmbientTemp : Inport
  Power : Inport  Port=2
  AmbTemp : Subsystem  mask{FP=Nominal, FT=0, FM=0}
      mask prompts: FP "Fault Profile:"; FT "Fault Injection Time:"; FM "Fault Magnitude/Slope/Bias/StuckAtValue"
      mask TypeOptions FP: Nominal | Abrupt | Incipient | Bias | StuckAt
      mask Display: image(imread('MSe.png','BackgroundColor',[1 1 1]))
  Ctheta : Subsystem  mask{C=96.176, IC=22, FP=Nominal, FT=0, FM=0}
      mask prompts: C "Value of C:"; IC "Initial Condition:"; FP "Fault Profile:"; FT "Fault Injection Time:"; FM "Fault Magnitude/Slope"
      mask TypeOptions FP: Nominal | Abrupt | Incipient
      mask Display: image(imread('C.png','BackgroundColor',[1 1 1]))
  ModAmbTemp : Subsystem
      mask Display: image(imread('fx.png','BackgroundColor',[1 1 1]))
  ModPs : Subsystem
      mask Display: image(imread('fx.png','BackgroundColor',[1 1 1]))
  OJ : Subsystem
      mask Display: image(imread('1.png','BackgroundColor',[1 1 1]))
  Rtheta : Subsystem  mask{R=1.5203, FP=Nominal, FT=0, FM=0}
      mask prompts: R "Value of R:"; FP "Fault Profile:"; FT "Fault Injection Time:"; FM "Fault Magnitude/Slope"
      mask TypeOptions FP: Nominal | Abrupt | Incipient
      mask Display: image(imread('R.png','BackgroundColor',[1 1 1]))
  SfPs : Subsystem  mask{FP=Nominal, FT=0, FM=0}
      mask prompts: FP "Fault Profile:"; FT "Fault Injection Time:"; FM "Fault Magnitude/Slope/Bias/StuckAtValue"
      mask TypeOptions FP: Nominal | Abrupt | Incipient | Bias | StuckAt
      mask Display: image(imread('MSf.png','BackgroundColor',[1 1 1]))
  theta : Subsystem
      mask Display: image(imread('0.png','BackgroundColor',[1 1 1]))
  Temp : Outport
  wires:
  SfPs/1(F) -> theta/1(u(1))
  theta/1(y(1)) -> SfPs/1(E)
  theta/2(y(2)) -> OJ/1(u(1))
  OJ/1(y(1)) -> theta/2(u(2))
  theta/3(y(3)) -> Ctheta/1(F)
  Ctheta/1(E) -> theta/3(u(3))
  OJ/2(y(2)) -> Rtheta/1(In)
  Rtheta/1(Out) -> OJ/2(u(2))
  AmbTemp/1(E) -> OJ/3(u(3))
  OJ/3(y(3)) -> AmbTemp/1(F)
  ModAmbTemp/1(y(1)) -> AmbTemp/2(Se)
  ModPs/1(y(1)) -> SfPs/2(Sf)
  Power/1 -> ModPs/1(u(1))
  AmbientTemp/1 -> ModAmbTemp/1(u(1))
  theta/4(O) -> Temp/1

== system VirtualADAPT/VirtualADAPTv1/PowerDistribution/ACLoadBank1/L1A/LGT6/LightBulb2/V ==
  u(1) : Inport
  u(2) : Inport  Port=2
  Adder : Sum  Inputs=-  SaturateOnIntegerOverflow=on  InputSameDT=on  OutDataTypeStr=Inherit: Same as first input
  Gain : Gain  Gain=-1  SaturateOnIntegerOverflow=on  OutDataTypeStr=Inherit: Same as input  ParamDataTypeStr=Inherit: Same as input
  y(1) : Outport
  y(2) : Outport  Port=2
  O : Outport  Port=3
  wires:
  Adder/1 -> Gain/1
  Gain/1 -> y(1)/1
  u(1)/1 -> y(2)/1, O/1
  u(2)/1 -> Adder/1

== system VirtualADAPT/VirtualADAPTv1/PowerDistribution/ACLoadBank1/L1A/LGT6/LightBulb3/ComputePower ==
  u(1) : Inport
  u(2) : Inport  Port=2
  Function : Fcn  Expr=abs(u(1)*u(2))
  Mux : Mux  Inputs=2
  y(1) : Outport
  wires:
  u(1)/1 -> Mux/1
  u(2)/1 -> Mux/2
  Mux/1 -> Function/1
  Function/1 -> y(1)/1

== system VirtualADAPT/VirtualADAPTv1/PowerDistribution/ACLoadBank1/L1A/LGT6/LightBulb3/I ==
  u(1) : Inport
  u(2) : Inport  Port=2
  Adder : Sum  Inputs=+  SaturateOnIntegerOverflow=on  InputSameDT=on  OutDataTypeStr=Inherit: Same as first input
  Gain : Gain  SaturateOnIntegerOverflow=on  OutDataTypeStr=Inherit: Same as input  ParamDataTypeStr=Inherit: Same as input
  y(1) : Outport
  y(2) : Outport  Port=2
  O : Outport  Port=3
  wires:
  Adder/1 -> Gain/1
  Gain/1 -> y(1)/1
  u(1)/1 -> y(2)/1, O/1
  u(2)/1 -> Adder/1

== system VirtualADAPT/VirtualADAPTv1/PowerDistribution/ACLoadBank1/L1A/LGT6/LightBulb3/R ==
  In : Inport
  Bond : Constant
  Computing R : Subsystem
  Fault Profile : Constant  Value=FP
  JuncDB : Constant  Value=2
  Junction Type : Constant  Value=0
  Ramp : simulink/Sources/Ramp  slope=FM  start=FT  X0=R
  Resistor Value : Constant  Value=R
  Step : Step  Time=FT  Before=R  After=120.5/(FM+120.5/R)  SampleTime=0
  Out : Outport
  R : Outport  Port=2
  wires:
  Computing R/1(Out1) -> Out/1
  In/1 -> Computing R/1(In1)
  Resistor Value/1 -> Computing R/2(RNominal)
  Fault Profile/1 -> Computing R/3(Fault Profile)
  Step/1 -> Computing R/4(Step)
  Ramp/1 -> Computing R/5(Ramp)
  JuncDB/1 -> Computing R/6(JuncDB)
  Bond/1 -> Computing R/7(Bond)
  Junction Type/1 -> Computing R/8(Junction Type)
  Computing R/2(Out2) -> R/1

== system VirtualADAPT/VirtualADAPTv1/PowerDistribution/ACLoadBank1/L1A/LGT6/LightBulb3/TemperatureModel ==
  AmbientTemp : Inport
  Power : Inport  Port=2
  AmbTemp : Subsystem  mask{FP=Nominal, FT=0, FM=0}
      mask prompts: FP "Fault Profile:"; FT "Fault Injection Time:"; FM "Fault Magnitude/Slope/Bias/StuckAtValue"
      mask TypeOptions FP: Nominal | Abrupt | Incipient | Bias | StuckAt
      mask Display: image(imread('MSe.png','BackgroundColor',[1 1 1]))
  Ctheta : Subsystem  mask{C=104.07, IC=22, FP=Nominal, FT=0, FM=0}
      mask prompts: C "Value of C:"; IC "Initial Condition:"; FP "Fault Profile:"; FT "Fault Injection Time:"; FM "Fault Magnitude/Slope"
      mask TypeOptions FP: Nominal | Abrupt | Incipient
      mask Display: image(imread('C.png','BackgroundColor',[1 1 1]))
  ModAmbTemp : Subsystem
      mask Display: image(imread('fx.png','BackgroundColor',[1 1 1]))
  ModPs : Subsystem
      mask Display: image(imread('fx.png','BackgroundColor',[1 1 1]))
  OJ : Subsystem
      mask Display: image(imread('1.png','BackgroundColor',[1 1 1]))
  Rtheta : Subsystem  mask{R=1.3978, FP=Nominal, FT=0, FM=0}
      mask prompts: R "Value of R:"; FP "Fault Profile:"; FT "Fault Injection Time:"; FM "Fault Magnitude/Slope"
      mask TypeOptions FP: Nominal | Abrupt | Incipient
      mask Display: image(imread('R.png','BackgroundColor',[1 1 1]))
  SfPs : Subsystem  mask{FP=Nominal, FT=0, FM=0}
      mask prompts: FP "Fault Profile:"; FT "Fault Injection Time:"; FM "Fault Magnitude/Slope/Bias/StuckAtValue"
      mask TypeOptions FP: Nominal | Abrupt | Incipient | Bias | StuckAt
      mask Display: image(imread('MSf.png','BackgroundColor',[1 1 1]))
  theta : Subsystem
      mask Display: image(imread('0.png','BackgroundColor',[1 1 1]))
  Temp : Outport
  wires:
  AmbTemp/1(E) -> OJ/1(u(1))
  OJ/1(y(1)) -> AmbTemp/1(F)
  theta/1(y(1)) -> Ctheta/1(F)
  Ctheta/1(E) -> theta/1(u(1))
  theta/2(y(2)) -> OJ/2(u(2))
  OJ/2(y(2)) -> theta/2(u(2))
  OJ/3(y(3)) -> Rtheta/1(In)
  Rtheta/1(Out) -> OJ/3(u(3))
  SfPs/1(F) -> theta/3(u(3))
  theta/3(y(3)) -> SfPs/1(E)
  ModPs/1(y(1)) -> SfPs/2(Sf)
  ModAmbTemp/1(y(1)) -> AmbTemp/2(Se)
  Power/1 -> ModPs/1(u(1))
  AmbientTemp/1 -> ModAmbTemp/1(u(1))
  theta/4(O) -> Temp/1

== system VirtualADAPT/VirtualADAPTv1/PowerDistribution/ACLoadBank1/L1A/LGT6/LightBulb3/V ==
  u(1) : Inport
  u(2) : Inport  Port=2
  Adder : Sum  Inputs=-  SaturateOnIntegerOverflow=on  InputSameDT=on  OutDataTypeStr=Inherit: Same as first input
  Gain : Gain  Gain=-1  SaturateOnIntegerOverflow=on  OutDataTypeStr=Inherit: Same as input  ParamDataTypeStr=Inherit: Same as input
  y(1) : Outport
  y(2) : Outport  Port=2
  O : Outport  Port=3
  wires:
  Adder/1 -> Gain/1
  u(2)/1 -> y(1)/1, O/1
  u(1)/1 -> Adder/1
  Gain/1 -> y(2)/1

== system VirtualADAPT/VirtualADAPTv1/PowerDistribution/ACLoadBank1/L1A/SensorLT500/Bias/Fault Inject ==
  Nominal : Inport
  Fault Profile : Inport  Port=2
  Step : Inport  Port=3
  Ramp : Inport  Port=4
  Bias : Inport  Port=5
  stuckAt : Inport  Port=6
  Switch1 : MultiPortSwitch  Inputs=5  SaturateOnIntegerOverflow=on  InputSameDT=on
  Out1 : Outport
  wires:
  Nominal/1 -> Switch1/2
  Fault Profile/1 -> Switch1/1
  Step/1 -> Switch1/3
  Ramp/1 -> Switch1/4
  Bias/1 -> Switch1/5
  stuckAt/1 -> Switch1/6
  Switch1/1 -> Out1/1

== system VirtualADAPT/VirtualADAPTv1/PowerDistribution/ACLoadBank1/L1A/SensorLT500/Gain/Fault Inject ==
  Nominal : Inport
  Fault Profile : Inport  Port=2
  Step : Inport  Port=3
  Ramp : Inport  Port=4
  Bias : Inport  Port=5
  stuckAt : Inport  Port=6
  Switch1 : MultiPortSwitch  Inputs=5  SaturateOnIntegerOverflow=on  InputSameDT=on
  Out1 : Outport
  wires:
  Nominal/1 -> Switch1/2
  Fault Profile/1 -> Switch1/1
  Step/1 -> Switch1/3
  Ramp/1 -> Switch1/4
  Bias/1 -> Switch1/5
  stuckAt/1 -> Switch1/6
  Switch1/1 -> Out1/1

== system VirtualADAPT/VirtualADAPTv1/PowerDistribution/ACLoadBank1/L1A/SensorLT500/MSe/Fault Inject ==
  Nominal : Inport
  Fault Profile : Inport  Port=2
  Step : Inport  Port=3
  Ramp : Inport  Port=4
  Bias : Inport  Port=5
  stuckAt : Inport  Port=6
  Switch1 : MultiPortSwitch  Inputs=5  SaturateOnIntegerOverflow=on  InputSameDT=on
  Out1 : Outport
  wires:
  Nominal/1 -> Switch1/2
  Fault Profile/1 -> Switch1/1
  Step/1 -> Switch1/3
  Ramp/1 -> Switch1/4
  Bias/1 -> Switch1/5
  stuckAt/1 -> Switch1/6
  Switch1/1 -> Out1/1

== system VirtualADAPT/VirtualADAPTv1/PowerDistribution/ACLoadBank1/L1A/SensorLT500/R/Computing R ==
  In1 : Inport
  RNominal : Inport  Port=2
  Fault Profile : Inport  Port=3
  Step : Inport  Port=4
  Ramp : Inport  Port=5
  JuncDB : Inport  Port=6
  Bond : Inport  Port=7
  Junction Type : Inport  Port=8
  CalcCausality : Fcn  Expr=(u(1) == u(2) && u(3) ==1) || (u(1) !=u(2) && u(3) == 0)
  CalcOutput : Fcn  Expr=(u(1)*u(2)^u(3))
  MinusOne : Constant  Value=-1
  Mux1 : Mux  Inputs=3
  Mux2 : Mux  Inputs=3
  One : Constant
  Switch1 : MultiPortSwitch  SaturateOnIntegerOverflow=on  InputSameDT=on
  Switch2 : MultiPortSwitch  DataPortOrder=Zero-based contiguous  Inputs=2  zeroidx=on  SaturateOnIntegerOverflow=on  InputSameDT=on
  Out1 : Outport
  Out2 : Outport  Port=2
  wires:
  In1/1 -> Mux2/1
  Fault Profile/1 -> Switch1/1
  RNominal/1 -> Switch1/2
  Step/1 -> Switch1/3
  Ramp/1 -> Switch1/4
  Switch1/1 -> Out2/1, Mux2/2
  JuncDB/1 -> Mux1/1
  Bond/1 -> Mux1/2
  Junction Type/1 -> Mux1/3
  Mux1/1 -> CalcCausality/1
  CalcCausality/1 -> Switch2/1
  One/1 -> Switch2/2
  MinusOne/1 -> Switch2/3
  Switch2/1 -> Mux2/3
  Mux2/1 -> CalcOutput/1
  CalcOutput/1 -> Out1/1

== system VirtualADAPT/VirtualADAPTv1/PowerDistribution/ACLoadBank1/L1A/SensorTE500/MSe/Fault Inject ==
  Nominal : Inport
  Fault Profile : Inport  Port=2
  Step : Inport  Port=3
  Ramp : Inport  Port=4
  Bias : Inport  Port=5
  stuckAt : Inport  Port=6
  Switch1 : MultiPortSwitch  Inputs=5  SaturateOnIntegerOverflow=on  InputSameDT=on
  Out1 : Outport
  wires:
  Nominal/1 -> Switch1/2
  Fault Profile/1 -> Switch1/1
  Step/1 -> Switch1/3
  Ramp/1 -> Switch1/4
  Bias/1 -> Switch1/5
  stuckAt/1 -> Switch1/6
  Switch1/1 -> Out1/1

== system VirtualADAPT/VirtualADAPTv1/PowerDistribution/ACLoadBank1/L1A/SensorTE500/R/Computing R ==
  In1 : Inport
  RNominal : Inport  Port=2
  Fault Profile : Inport  Port=3
  Step : Inport  Port=4
  Ramp : Inport  Port=5
  JuncDB : Inport  Port=6
  Bond : Inport  Port=7
  Junction Type : Inport  Port=8
  CalcCausality : Fcn  Expr=(u(1) == u(2) && u(3) ==1) || (u(1) !=u(2) && u(3) == 0)
  CalcOutput : Fcn  Expr=(u(1)*u(2)^u(3))
  MinusOne : Constant  Value=-1
  Mux1 : Mux  Inputs=3
  Mux2 : Mux  Inputs=3
  One : Constant
  Switch1 : MultiPortSwitch  SaturateOnIntegerOverflow=on  InputSameDT=on
  Switch2 : MultiPortSwitch  DataPortOrder=Zero-based contiguous  Inputs=2  zeroidx=on  SaturateOnIntegerOverflow=on  InputSameDT=on
  Out1 : Outport
  Out2 : Outport  Port=2
  wires:
  In1/1 -> Mux2/1
  Fault Profile/1 -> Switch1/1
  RNominal/1 -> Switch1/2
  Step/1 -> Switch1/3
  Ramp/1 -> Switch1/4
  Switch1/1 -> Out2/1, Mux2/2
  JuncDB/1 -> Mux1/1
  Bond/1 -> Mux1/2
  Junction Type/1 -> Mux1/3
  Mux1/1 -> CalcCausality/1
  CalcCausality/1 -> Switch2/1
  One/1 -> Switch2/2
  MinusOne/1 -> Switch2/3
  Switch2/1 -> Mux2/3
  Mux2/1 -> CalcOutput/1
  CalcOutput/1 -> Out1/1

== system VirtualADAPT/VirtualADAPTv1/PowerDistribution/ACLoadBank1/L1A/SensorTE500/TempBias/Fault Inject ==
  Nominal : Inport
  Fault Profile : Inport  Port=2
  Step : Inport  Port=3
  Ramp : Inport  Port=4
  Bias : Inport  Port=5
  stuckAt : Inport  Port=6
  Switch1 : MultiPortSwitch  Inputs=5  SaturateOnIntegerOverflow=on  InputSameDT=on
  Out1 : Outport
  wires:
  Nominal/1 -> Switch1/2
  Fault Profile/1 -> Switch1/1
  Step/1 -> Switch1/3
  Ramp/1 -> Switch1/4
  Bias/1 -> Switch1/5
  stuckAt/1 -> Switch1/6
  Switch1/1 -> Out1/1

== system VirtualADAPT/VirtualADAPTv1/PowerDistribution/ACLoadBank1/L1A/SensorTE501/MSe/Fault Inject ==
  Nominal : Inport
  Fault Profile : Inport  Port=2
  Step : Inport  Port=3
  Ramp : Inport  Port=4
  Bias : Inport  Port=5
  stuckAt : Inport  Port=6
  Switch1 : MultiPortSwitch  Inputs=5  SaturateOnIntegerOverflow=on  InputSameDT=on
  Out1 : Outport
  wires:
  Nominal/1 -> Switch1/2
  Fault Profile/1 -> Switch1/1
  Step/1 -> Switch1/3
  Ramp/1 -> Switch1/4
  Bias/1 -> Switch1/5
  stuckAt/1 -> Switch1/6
  Switch1/1 -> Out1/1

== system VirtualADAPT/VirtualADAPTv1/PowerDistribution/ACLoadBank1/L1A/SensorTE501/R/Computing R ==
  In1 : Inport
  RNominal : Inport  Port=2
  Fault Profile : Inport  Port=3
  Step : Inport  Port=4
  Ramp : Inport  Port=5
  JuncDB : Inport  Port=6
  Bond : Inport  Port=7
  Junction Type : Inport  Port=8
  CalcCausality : Fcn  Expr=(u(1) == u(2) && u(3) ==1) || (u(1) !=u(2) && u(3) == 0)
  CalcOutput : Fcn  Expr=(u(1)*u(2)^u(3))
  MinusOne : Constant  Value=-1
  Mux1 : Mux  Inputs=3
  Mux2 : Mux  Inputs=3
  One : Constant
  Switch1 : MultiPortSwitch  SaturateOnIntegerOverflow=on  InputSameDT=on
  Switch2 : MultiPortSwitch  DataPortOrder=Zero-based contiguous  Inputs=2  zeroidx=on  SaturateOnIntegerOverflow=on  InputSameDT=on
  Out1 : Outport
  Out2 : Outport  Port=2
  wires:
  In1/1 -> Mux2/1
  Fault Profile/1 -> Switch1/1
  RNominal/1 -> Switch1/2
  Step/1 -> Switch1/3
  Ramp/1 -> Switch1/4
  Switch1/1 -> Out2/1, Mux2/2
  JuncDB/1 -> Mux1/1
  Bond/1 -> Mux1/2
  Junction Type/1 -> Mux1/3
  Mux1/1 -> CalcCausality/1
  CalcCausality/1 -> Switch2/1
  One/1 -> Switch2/2
  MinusOne/1 -> Switch2/3
  Switch2/1 -> Mux2/3
  Mux2/1 -> CalcOutput/1
  CalcOutput/1 -> Out1/1

== system VirtualADAPT/VirtualADAPTv1/PowerDistribution/ACLoadBank1/L1A/SensorTE501/TempBias/Fault Inject ==
  Nominal : Inport
  Fault Profile : Inport  Port=2
  Step : Inport  Port=3
  Ramp : Inport  Port=4
  Bias : Inport  Port=5
  stuckAt : Inport  Port=6
  Switch1 : MultiPortSwitch  Inputs=5  SaturateOnIntegerOverflow=on  InputSameDT=on
  Out1 : Outport
  wires:
  Nominal/1 -> Switch1/2
  Fault Profile/1 -> Switch1/1
  Step/1 -> Switch1/3
  Ramp/1 -> Switch1/4
  Bias/1 -> Switch1/5
  stuckAt/1 -> Switch1/6
  Switch1/1 -> Out1/1

== system VirtualADAPT/VirtualADAPTv1/PowerDistribution/ACLoadBank1/L1A/SensorTE502/MSe/Fault Inject ==
  Nominal : Inport
  Fault Profile : Inport  Port=2
  Step : Inport  Port=3
  Ramp : Inport  Port=4
  Bias : Inport  Port=5
  stuckAt : Inport  Port=6
  Switch1 : MultiPortSwitch  Inputs=5  SaturateOnIntegerOverflow=on  InputSameDT=on
  Out1 : Outport
  wires:
  Nominal/1 -> Switch1/2
  Fault Profile/1 -> Switch1/1
  Step/1 -> Switch1/3
  Ramp/1 -> Switch1/4
  Bias/1 -> Switch1/5
  stuckAt/1 -> Switch1/6
  Switch1/1 -> Out1/1

== system VirtualADAPT/VirtualADAPTv1/PowerDistribution/ACLoadBank1/L1A/SensorTE502/R/Computing R ==
  In1 : Inport
  RNominal : Inport  Port=2
  Fault Profile : Inport  Port=3
  Step : Inport  Port=4
  Ramp : Inport  Port=5
  JuncDB : Inport  Port=6
  Bond : Inport  Port=7
  Junction Type : Inport  Port=8
  CalcCausality : Fcn  Expr=(u(1) == u(2) && u(3) ==1) || (u(1) !=u(2) && u(3) == 0)
  CalcOutput : Fcn  Expr=(u(1)*u(2)^u(3))
  MinusOne : Constant  Value=-1
  Mux1 : Mux  Inputs=3
  Mux2 : Mux  Inputs=3
  One : Constant
  Switch1 : MultiPortSwitch  SaturateOnIntegerOverflow=on  InputSameDT=on
  Switch2 : MultiPortSwitch  DataPortOrder=Zero-based contiguous  Inputs=2  zeroidx=on  SaturateOnIntegerOverflow=on  InputSameDT=on
  Out1 : Outport
  Out2 : Outport  Port=2
  wires:
  In1/1 -> Mux2/1
  Fault Profile/1 -> Switch1/1
  RNominal/1 -> Switch1/2
  Step/1 -> Switch1/3
  Ramp/1 -> Switch1/4
  Switch1/1 -> Out2/1, Mux2/2
  JuncDB/1 -> Mux1/1
  Bond/1 -> Mux1/2
  Junction Type/1 -> Mux1/3
  Mux1/1 -> CalcCausality/1
  CalcCausality/1 -> Switch2/1
  One/1 -> Switch2/2
  MinusOne/1 -> Switch2/3
  Switch2/1 -> Mux2/3
  Mux2/1 -> CalcOutput/1
  CalcOutput/1 -> Out1/1

== system VirtualADAPT/VirtualADAPTv1/PowerDistribution/ACLoadBank1/L1A/SensorTE502/TempBias/Fault Inject ==
  Nominal : Inport
  Fault Profile : Inport  Port=2
  Step : Inport  Port=3
  Ramp : Inport  Port=4
  Bias : Inport  Port=5
  stuckAt : Inport  Port=6
  Switch1 : MultiPortSwitch  Inputs=5  SaturateOnIntegerOverflow=on  InputSameDT=on
  Out1 : Outport
  wires:
  Nominal/1 -> Switch1/2
  Fault Profile/1 -> Switch1/1
  Step/1 -> Switch1/3
  Ramp/1 -> Switch1/4
  Bias/1 -> Switch1/5
  stuckAt/1 -> Switch1/6
  Switch1/1 -> Out1/1

== system VirtualADAPT/VirtualADAPTv1/PowerDistribution/ACLoadBank1/L1B/FAN1/Friction/Computing R ==
  In1 : Inport
  RNominal : Inport  Port=2
  Fault Profile : Inport  Port=3
  Step : Inport  Port=4
  Ramp : Inport  Port=5
  JuncDB : Inport  Port=6
  Bond : Inport  Port=7
  Junction Type : Inport  Port=8
  CalcCausality : Fcn  Expr=(u(1) == u(2) && u(3) ==1) || (u(1) !=u(2) && u(3) == 0)
  CalcOutput : Fcn  Expr=(u(1)*u(2)^u(3))
  MinusOne : Constant  Value=-1
  Mux1 : Mux  Inputs=3
  Mux2 : Mux  Inputs=3
  One : Constant
  Switch1 : MultiPortSwitch  SaturateOnIntegerOverflow=on  InputSameDT=on
  Switch2 : MultiPortSwitch  DataPortOrder=Zero-based contiguous  Inputs=2  zeroidx=on  SaturateOnIntegerOverflow=on  InputSameDT=on
  Out1 : Outport
  Out2 : Outport  Port=2
  wires:
  In1/1 -> Mux2/1
  Fault Profile/1 -> Switch1/1
  RNominal/1 -> Switch1/2
  Step/1 -> Switch1/3
  Ramp/1 -> Switch1/4
  Switch1/1 -> Out2/1, Mux2/2
  JuncDB/1 -> Mux1/1
  Bond/1 -> Mux1/2
  Junction Type/1 -> Mux1/3
  Mux1/1 -> CalcCausality/1
  CalcCausality/1 -> Switch2/1
  One/1 -> Switch2/2
  MinusOne/1 -> Switch2/3
  Switch2/1 -> Mux2/3
  Mux2/1 -> CalcOutput/1
  CalcOutput/1 -> Out1/1

== system VirtualADAPT/VirtualADAPTv1/PowerDistribution/ACLoadBank1/L1B/FAN1/GY/Fault Inject ==
  Nominal : Inport
  Fault Profile : Inport  Port=2
  Step : Inport  Port=3
  Ramp : Inport  Port=4
  Bias : Inport  Port=5
  stuckAt : Inport  Port=6
  Switch1 : MultiPortSwitch  Inputs=5  SaturateOnIntegerOverflow=on  InputSameDT=on
  Out1 : Outport
  wires:
  Nominal/1 -> Switch1/2
  Fault Profile/1 -> Switch1/1
  Step/1 -> Switch1/3
  Ramp/1 -> Switch1/4
  Bias/1 -> Switch1/5
  stuckAt/1 -> Switch1/6
  Switch1/1 -> Out1/1

== system VirtualADAPT/VirtualADAPTv1/PowerDistribution/ACLoadBank1/L1B/FAN1/Inductance/Fault Inject ==
  Nominal : Inport
  Fault Profile : Inport  Port=2
  Step : Inport  Port=3
  Ramp : Inport  Port=4
  Bias : Inport  Port=5
  stuckAt : Inport  Port=6
  Switch1 : MultiPortSwitch  Inputs=5  SaturateOnIntegerOverflow=on  InputSameDT=on
  Out1 : Outport
  wires:
  Nominal/1 -> Switch1/2
  Fault Profile/1 -> Switch1/1
  Step/1 -> Switch1/3
  Ramp/1 -> Switch1/4
  Bias/1 -> Switch1/5
  stuckAt/1 -> Switch1/6
  Switch1/1 -> Out1/1

== system VirtualADAPT/VirtualADAPTv1/PowerDistribution/ACLoadBank1/L1B/FAN1/Inertia/Fault Inject ==
  Nominal : Inport
  Fault Profile : Inport  Port=2
  Step : Inport  Port=3
  Ramp : Inport  Port=4
  Bias : Inport  Port=5
  stuckAt : Inport  Port=6
  Switch1 : MultiPortSwitch  Inputs=5  SaturateOnIntegerOverflow=on  InputSameDT=on
  Out1 : Outport
  wires:
  Nominal/1 -> Switch1/2
  Fault Profile/1 -> Switch1/1
  Step/1 -> Switch1/3
  Ramp/1 -> Switch1/4
  Bias/1 -> Switch1/5
  stuckAt/1 -> Switch1/6
  Switch1/1 -> Out1/1

== system VirtualADAPT/VirtualADAPTv1/PowerDistribution/ACLoadBank1/L1B/FAN1/Resistance/Computing R ==
  In1 : Inport
  RNominal : Inport  Port=2
  Fault Profile : Inport  Port=3
  Step : Inport  Port=4
  Ramp : Inport  Port=5
  JuncDB : Inport  Port=6
  Bond : Inport  Port=7
  Junction Type : Inport  Port=8
  CalcCausality : Fcn  Expr=(u(1) == u(2) && u(3) ==1) || (u(1) !=u(2) && u(3) == 0)
  CalcOutput : Fcn  Expr=(u(1)*u(2)^u(3))
  MinusOne : Constant  Value=-1
  Mux1 : Mux  Inputs=3
  Mux2 : Mux  Inputs=3
  One : Constant
  Switch1 : MultiPortSwitch  SaturateOnIntegerOverflow=on  InputSameDT=on
  Switch2 : MultiPortSwitch  DataPortOrder=Zero-based contiguous  Inputs=2  zeroidx=on  SaturateOnIntegerOverflow=on  InputSameDT=on
  Out1 : Outport
  Out2 : Outport  Port=2
  wires:
  In1/1 -> Mux2/1
  Fault Profile/1 -> Switch1/1
  RNominal/1 -> Switch1/2
  Step/1 -> Switch1/3
  Ramp/1 -> Switch1/4
  Switch1/1 -> Out2/1, Mux2/2
  JuncDB/1 -> Mux1/1
  Bond/1 -> Mux1/2
  Junction Type/1 -> Mux1/3
  Mux1/1 -> CalcCausality/1
  CalcCausality/1 -> Switch2/1
  One/1 -> Switch2/2
  MinusOne/1 -> Switch2/3
  Switch2/1 -> Mux2/3
  Mux2/1 -> CalcOutput/1
  CalcOutput/1 -> Out1/1

== system VirtualADAPT/VirtualADAPTv1/PowerDistribution/ACLoadBank1/L1B/SensorST515/Bias/Fault Inject ==
  Nominal : Inport
  Fault Profile : Inport  Port=2
  Step : Inport  Port=3
  Ramp : Inport  Port=4
  Bias : Inport  Port=5
  stuckAt : Inport  Port=6
  Switch1 : MultiPortSwitch  Inputs=5  SaturateOnIntegerOverflow=on  InputSameDT=on
  Out1 : Outport
  wires:
  Nominal/1 -> Switch1/2
  Fault Profile/1 -> Switch1/1
  Step/1 -> Switch1/3
  Ramp/1 -> Switch1/4
  Bias/1 -> Switch1/5
  stuckAt/1 -> Switch1/6
  Switch1/1 -> Out1/1
